simulink model "MCVT_Integration"  (R2020a)
settings: Solver=ode8  StartTime=0.0  StopTime=T_end  FixedStep=dt  RelTol=1e-3  EnableMultiTasking=on
values from neighbouring files (symbols this model uses):
  assumed_power_voltage = 1.25e2    (= 125)    [MCVT_Input_File.m]    (also in MCVT_Run_QuickCheck.m)
  battery_power_toggle = 1    [MCVT_Input_File.m]    (also in MCVT_Run_QuickCheck.m)
  battery_trickle_charge_param = 0.1    [MCVT_Input_File.m]    (also in MCVT_Run_QuickCheck.m)
  battery_trickle_leakage_param = 0.01    [MCVT_Input_File.m]    (also in MCVT_Run_QuickCheck.m)
  damage_case_id = 3    [MCVT_Input_File.m]    (also in MCVT_Run_QuickCheck.m = 1)
  dt = 1/fs    (= 0.001)    [MCVT_Run_QuickCheck.m]    (also in MCVT_Sim_Set.m)
  dt_2 = 1/fs_2    (= 0.001)    [MCVT_Run_QuickCheck.m]    (also in MCVT_Sim_Set.m)
  dt_3 = 1/fs_3    (= 0.001)    [MCVT_Run_QuickCheck.m]    (also in MCVT_Sim_Set.m)
  dt_5 = 1/fs_5    (= 0.005)    [MCVT_Run_QuickCheck.m]    (also in MCVT_Sim_Set.m)
  dt_8 = 1/fs_8    (= 0.05)    [MCVT_Run_QuickCheck.m]    (also in MCVT_Sim_Set.m)
  dt_9 = 1/fs_9    (= 0.001)    [MCVT_Run_QuickCheck.m]    (also in MCVT_Sim_Set.m)
  dust_met_duration = 3    [MCVT_Input_File.m]    (also in MCVT_Run_QuickCheck.m = 2)
  ECLSS_indoor_set_temperature = 298.15    [MCVT_Input_File.m]    (also in MCVT_Run_QuickCheck.m)
  energy_store_max_o = 150    [MCVT_Input_File.m]    (also in MCVT_Run_QuickCheck.m)
  energy_store_o = 120    [MCVT_Input_File.m]    (also in MCVT_Run_QuickCheck.m)
  fs = 1000    [MCVT_Run_QuickCheck.m]    (also in MCVT_Sim_Set.m)
  gain_charge_param = 1    [MCVT_Input_File.m]    (also in MCVT_Run_QuickCheck.m)
  ground_temperature = 373    [MCVT_Habitat_Design_Input_File.m]    (also in MCVT_Run_QuickCheck.m)
  I_max_charge = 800    [MCVT_Input_File.m]    (also in MCVT_Run_QuickCheck.m)
  I_max_discharge = 800    [MCVT_Input_File.m]    (also in MCVT_Run_QuickCheck.m)
  initial_pressure = 1e5    (= 100000)    [MCVT_Input_File.m]    (also in MCVT_Run_QuickCheck.m)
  initial_temperature = 295    [MCVT_Input_File.m]    (also in MCVT_Run_QuickCheck.m)
  interior_leak_rate = 0.00415    [MCVT_Input_File.m]    (also in MCVT_Run_QuickCheck.m)
  launch_dust_accum_start_time = 10    [MCVT_Input_File.m]    (also in MCVT_Run_QuickCheck.m = 2)
  launch_dust_accum_stop_time = 12    [MCVT_Input_File.m]    (also in MCVT_Run_QuickCheck.m = 3)
  meteorite_impact_time = 2    [MCVT_Input_File.m]    (also in MCVT_Run_QuickCheck.m = 1)
  nuclear_power_toggle = 1    [MCVT_Input_File.m]    (also in MCVT_Run_QuickCheck.m)
  print_interval = 30    [MCVT_Input_File.m]
  SOC_lower_thres = 0.5    [MCVT_Input_File.m]    (also in MCVT_Run_QuickCheck.m)
  SOC_upper_thres = 0.9    [MCVT_Input_File.m]    (also in MCVT_Run_QuickCheck.m)
  solar_power_toggle = 1    [MCVT_Input_File.m]    (also in MCVT_Run_QuickCheck.m)
  space_temperature = 4    [MCVT_Habitat_Design_Input_File.m]    (also in MCVT_Run_QuickCheck.m)
  Start_angle = 90    [MCVT_Input_File.m]    (also in MCVT_Run_QuickCheck.m)
undefined symbols (not defined in the file or in its folder): A_flow_restrictor, ACTIVITY_LOOKUP_TABLE, air, Aref, Bmap, Bmap2, Bmap3, comms, DiscIntNeverNeededParam, DmgSPL, dust_parameters, ECLSS_dust_damage_repair_time, ECLSS_heater_capacity, ECLSS_IPS_restrictor, ECLSS_IPS_setpointPressure, ECLSS_paint_damage_repair_time, ECLSS_radiatorLoop_discreteNumber, ECLSS_Vst, emptyActivity, expected_heat_add, FI, habitat, HIEM2SMM, HIEM2STM, HIEMDamageElements, impact_numbering, initial_SPLT_damage_index, input_air, input_Cond, input_CP, input_discretization, input_EV, input_Evap, input_radiatorLoop, K_ECLSS_dust_single, K_ECLSS_paint_single, K_Structure_damage_single, Kref, kW_to_W, meteoroidDustDep, mFRF, ndelays, nominalDustDep, noverlap, nuclear_toggle_gain, output_limits, panel_paint0, Ps0, reference_temperature, RepairTime_ECLSS_factor, Sensor_Location_SMM, SMM_T0, SMM_T1, SMM_T2, SMM_T3, SMM_T4, SMM_T4_0, SMM_T4_1, SMM_T4_2, solar_FDD_K ... (+73)
libraries: neural x350, simulink x39, xpcutilitieslib x7, xpcrtudplib x7, dspstat3 x1
(part 32 of 43)

== system MCVT_Integration/Sys2 - Structural System/Sys2_Structural_System/Fault Detection and Diagnosis (FDD) with Binary Classifer/Subsystem/Enabled Subsystem3/Pattern Recognition Neural Network1/Layer 2 ==
  "a{1} " : Inport  PortDimensions=30  SampleTime=0.001
      mask Display: e = 0.06;c1 = [120 230 180]/255;c2 = ([120 230 180]+0)/(256+128);c3 = ([120 230 180]+128)/(256+128);patch([0 1 1 0],[0 0 1 1],c1);patch([0 1 1 1-e 1-e 0+e],[0 0 1 1-e 0+e 0+e],c2);patch([0 0 1 1-e 0+e 0+e],[0 1 1 1-e 1-e 0+e],c3);plot([0 1 1 0 0],[0 0 1 1 0]);
  Delays 1 : Subsystem
      mask Display: e = 0.06;c1 = [120 150 230]/255;c2 = ([120 150 230]+0)/383;c3 = ([120 150 230]+128)/383;patch([0 1 1 0],[0 0 1 1],c1);patch([0 1 1 1-e 1-e 0+e],[0 0 1 1-e 0+e 0+e],c2);patch([0 0 1 1-e 0+e 0+e],[0 1 1 1-e 1-e 0+e],c3);plot([0 1 1 0 0],[0 0 1 1 0]);disp('0');
  "LW{2,1}" : Subsystem
      mask Display: e = 0.06;c1 = [120 150 230]/255;c2 = ([120 150 230]+0)/383;c3 = ([120 150 230]+128)/383;patch([0 1 1 0],[0 0 1 1],c1);patch([0 1 1 1-e 1-e 0+e],[0 0 1 1-e 0+e 0+e],c2);patch([0 0 1 1-e 0+e 0+e],[0 1 1 1-e 1-e 0+e],c3);plot([0 1 1 0 0],[0 0 1 1 0]);disp('W');
  "b{2}" : Constant  Value=[-1.5675181578718626607127362149185501039028167724609375;-1.3718445952097766404875756052206270396709442138671875;1.0992311692557044100482244175509549677371978759765625;1.1150228436739049175940863278810866177082061767578125;0.85025790232238362964523048503906466066837310791015625;-0.6689209990231959057638277954538352787494659423828125;-0.54887147293178861762186215855763293802738189697265625;-0.43766829067725743218630896080867387354373931884765625;-0.208936085733707643807832710081129334867000579833984375;0.057764402550326297836047473310827626846730709075927734375;-0.1666376935928840230527470112065202556550502777099609375;0.278159185776559070291114039719104766845703125;0.372658163201040559808774332850589416921138763427734375;0.5401695979973555328257361907162703573703765869140625;-0.714168735853778269273561818408779799938201904296875;0.8295230504828852158283325479715131223201751708984375;0.95406994019251578631468646563007496297359466552734375;-1.2131549221309152475356540890061296522617340087890625;1.267271803453536183070582410437054932117462158203125;-1.4500424122772075374854239271371625363826751708984375]
      mask Display: e = 0.06;c1 = [120 150 230]/255;c2 = ([120 150 230]+0)/383;c3 = ([120 150 230]+128)/383;patch([0 1 1 0],[0 0 1 1],c1);patch([0 1 1 1-e 1-e 0+e],[0 0 1 1-e 0+e 0+e],c2);patch([0 0 1 1-e 0+e 0+e],[0 1 1 1-e 1-e 0+e],c3);plot([0 1 1 0 0],[0 0 1 1 0]);disp('b');
  netsum : Sum  Inputs=2  InputSameDT=on  SaturateOnIntegerOverflow=on  OutDataTypeStr=Inherit: Same as first input
      mask Display: e = 0.06;c1 = [120 150 230]/255;c2 = ([120 150 230]+0)/383;c3 = ([120 150 230]+128)/383;patch([0 1 1 0],[0 0 1 1],c1);patch([0 1 1 1-e 1-e 0+e],[0 0 1 1-e 0+e 0+e],c2);patch([0 0 1 1-e 0+e 0+e],[0 1 1 1-e 1-e 0+e],c3);plot([0 1 1 0 0],[0 0 1 1 0]);disp('+');
  tansig : neural/Transfer Functions/tansig
  "a{2}" : Outport  InitialOutput=[0;0;0;0;0;0;0;0;0;0;0;0;0;0;0;0;0;0;0;0]
      mask Display: e = 0.06;c1 = [120 230 180]/255;c2 = ([120 230 180]+0)/(256+128);c3 = ([120 230 180]+128)/(256+128);patch([0 1 1 0],[0 0 1 1],c1);patch([0 1 1 1-e 1-e 0+e],[0 0 1 1-e 0+e 0+e],c2);patch([0 0 1 1-e 0+e 0+e],[0 1 1 1-e 1-e 0+e],c3);plot([0 1 1 0 0],[0 0 1 1 0]);
  wires:
  "a{1} "/1 -> Delays 1/1("p{2}")
  Delays 1/1("pd{2,1}") -> "LW{2,1}"/1("ad{2,1}")
  "LW{2,1}"/1("lz{2,1}") -> netsum/1
  "b{2}"/1 -> netsum/2
  netsum/1 -> tansig/1
  tansig/1 -> "a{2}"/1

== system MCVT_Integration/Sys2 - Structural System/Sys2_Structural_System/Fault Detection and Diagnosis (FDD) with Binary Classifer/Subsystem/Enabled Subsystem3/Pattern Recognition Neural Network1/Layer 3 ==
  "a{2} " : Inport  PortDimensions=20  SampleTime=0.001
      mask Display: e = 0.06;c1 = [120 230 180]/255;c2 = ([120 230 180]+0)/(256+128);c3 = ([120 230 180]+128)/(256+128);patch([0 1 1 0],[0 0 1 1],c1);patch([0 1 1 1-e 1-e 0+e],[0 0 1 1-e 0+e 0+e],c2);patch([0 0 1 1-e 0+e 0+e],[0 1 1 1-e 1-e 0+e],c3);plot([0 1 1 0 0],[0 0 1 1 0]);
  Delays 1 : Subsystem
      mask Display: e = 0.06;c1 = [120 150 230]/255;c2 = ([120 150 230]+0)/383;c3 = ([120 150 230]+128)/383;patch([0 1 1 0],[0 0 1 1],c1);patch([0 1 1 1-e 1-e 0+e],[0 0 1 1-e 0+e 0+e],c2);patch([0 0 1 1-e 0+e 0+e],[0 1 1 1-e 1-e 0+e],c3);plot([0 1 1 0 0],[0 0 1 1 0]);disp('0');
  "LW{3,2}" : Subsystem
      mask Display: e = 0.06;c1 = [120 150 230]/255;c2 = ([120 150 230]+0)/383;c3 = ([120 150 230]+128)/383;patch([0 1 1 0],[0 0 1 1],c1);patch([0 1 1 1-e 1-e 0+e],[0 0 1 1-e 0+e 0+e],c2);patch([0 0 1 1-e 0+e 0+e],[0 1 1 1-e 1-e 0+e],c3);plot([0 1 1 0 0],[0 0 1 1 0]);disp('W');
  "b{3}" : Constant  Value=[1.461288307463145752507216457161121070384979248046875;1.158168800422737287902918978943489491939544677734375;0.82605315684820423971501668347627855837345123291015625;-0.4114092128700901351834318120381794869899749755859375;-0.397272642706039402771267532443744130432605743408203125;-0.16670204583020098443313372627017088234424591064453125;0.52460758887335756650571738646249286830425262451171875;-0.83480056037617156317054423197987489402294158935546875;-1.258774443942892506953512565814889967441558837890625;1.7012363464620114950065499215270392596721649169921875]
      mask Display: e = 0.06;c1 = [120 150 230]/255;c2 = ([120 150 230]+0)/383;c3 = ([120 150 230]+128)/383;patch([0 1 1 0],[0 0 1 1],c1);patch([0 1 1 1-e 1-e 0+e],[0 0 1 1-e 0+e 0+e],c2);patch([0 0 1 1-e 0+e 0+e],[0 1 1 1-e 1-e 0+e],c3);plot([0 1 1 0 0],[0 0 1 1 0]);disp('b');
  netsum : Sum  Inputs=2  InputSameDT=on  SaturateOnIntegerOverflow=on  OutDataTypeStr=Inherit: Same as first input
      mask Display: e = 0.06;c1 = [120 150 230]/255;c2 = ([120 150 230]+0)/383;c3 = ([120 150 230]+128)/383;patch([0 1 1 0],[0 0 1 1],c1);patch([0 1 1 1-e 1-e 0+e],[0 0 1 1-e 0+e 0+e],c2);patch([0 0 1 1-e 0+e 0+e],[0 1 1 1-e 1-e 0+e],c3);plot([0 1 1 0 0],[0 0 1 1 0]);disp('+');
  tansig : neural/Transfer Functions/tansig
  "a{3}" : Outport  InitialOutput=[0;0;0;0;0;0;0;0;0;0]
      mask Display: e = 0.06;c1 = [120 230 180]/255;c2 = ([120 230 180]+0)/(256+128);c3 = ([120 230 180]+128)/(256+128);patch([0 1 1 0],[0 0 1 1],c1);patch([0 1 1 1-e 1-e 0+e],[0 0 1 1-e 0+e 0+e],c2);patch([0 0 1 1-e 0+e 0+e],[0 1 1 1-e 1-e 0+e],c3);plot([0 1 1 0 0],[0 0 1 1 0]);
  wires:
  "a{2} "/1 -> Delays 1/1("p{3}")
  Delays 1/1("pd{3,2}") -> "LW{3,2}"/1("ad{3,2}")
  "LW{3,2}"/1("lz{3,2}") -> netsum/1
  "b{3}"/1 -> netsum/2
  netsum/1 -> tansig/1
  tansig/1 -> "a{3}"/1

== system MCVT_Integration/Sys2 - Structural System/Sys2_Structural_System/Fault Detection and Diagnosis (FDD) with Binary Classifer/Subsystem/Enabled Subsystem3/Pattern Recognition Neural Network1/Layer 4 ==
  "a{3} " : Inport  PortDimensions=10  SampleTime=0.001
      mask Display: e = 0.06;c1 = [120 230 180]/255;c2 = ([120 230 180]+0)/(256+128);c3 = ([120 230 180]+128)/(256+128);patch([0 1 1 0],[0 0 1 1],c1);patch([0 1 1 1-e 1-e 0+e],[0 0 1 1-e 0+e 0+e],c2);patch([0 0 1 1-e 0+e 0+e],[0 1 1 1-e 1-e 0+e],c3);plot([0 1 1 0 0],[0 0 1 1 0]);
  Delays 1 : Subsystem
      mask Display: e = 0.06;c1 = [120 150 230]/255;c2 = ([120 150 230]+0)/383;c3 = ([120 150 230]+128)/383;patch([0 1 1 0],[0 0 1 1],c1);patch([0 1 1 1-e 1-e 0+e],[0 0 1 1-e 0+e 0+e],c2);patch([0 0 1 1-e 0+e 0+e],[0 1 1 1-e 1-e 0+e],c3);plot([0 1 1 0 0],[0 0 1 1 0]);disp('0');
  "LW{4,3}" : Subsystem
      mask Display: e = 0.06;c1 = [120 150 230]/255;c2 = ([120 150 230]+0)/383;c3 = ([120 150 230]+128)/383;patch([0 1 1 0],[0 0 1 1],c1);patch([0 1 1 1-e 1-e 0+e],[0 0 1 1-e 0+e 0+e],c2);patch([0 0 1 1-e 0+e 0+e],[0 1 1 1-e 1-e 0+e],c3);plot([0 1 1 0 0],[0 0 1 1 0]);disp('W');
  "b{4}" : Constant  Value=[-0.08562532905421325890582551210172823630273342132568359375;0.8902934228766004043365001052734442055225372314453125;-0.8595801172941406331773350757430307567119598388671875;-0.1235614075244175769086041327682323753833770751953125;1.164751385274076067588566729682497680187225341796875]
      mask Display: e = 0.06;c1 = [120 150 230]/255;c2 = ([120 150 230]+0)/383;c3 = ([120 150 230]+128)/383;patch([0 1 1 0],[0 0 1 1],c1);patch([0 1 1 1-e 1-e 0+e],[0 0 1 1-e 0+e 0+e],c2);patch([0 0 1 1-e 0+e 0+e],[0 1 1 1-e 1-e 0+e],c3);plot([0 1 1 0 0],[0 0 1 1 0]);disp('b');
  netsum : Sum  Inputs=2  InputSameDT=on  SaturateOnIntegerOverflow=on  OutDataTypeStr=Inherit: Same as first input
      mask Display: e = 0.06;c1 = [120 150 230]/255;c2 = ([120 150 230]+0)/383;c3 = ([120 150 230]+128)/383;patch([0 1 1 0],[0 0 1 1],c1);patch([0 1 1 1-e 1-e 0+e],[0 0 1 1-e 0+e 0+e],c2);patch([0 0 1 1-e 0+e 0+e],[0 1 1 1-e 1-e 0+e],c3);plot([0 1 1 0 0],[0 0 1 1 0]);disp('+');
  softmax : neural/Transfer Functions/softmax
      settings: SourceProductBaseCode=NN
  "a{4}" : Outport  InitialOutput=[0;0;0;0;0]
      mask Display: e = 0.06;c1 = [120 230 180]/255;c2 = ([120 230 180]+0)/(256+128);c3 = ([120 230 180]+128)/(256+128);patch([0 1 1 0],[0 0 1 1],c1);patch([0 1 1 1-e 1-e 0+e],[0 0 1 1-e 0+e 0+e],c2);patch([0 0 1 1-e 0+e 0+e],[0 1 1 1-e 1-e 0+e],c3);plot([0 1 1 0 0],[0 0 1 1 0]);
  wires:
  "a{3} "/1 -> Delays 1/1("p{4}")
  Delays 1/1("pd{4,3}") -> "LW{4,3}"/1("ad{4,3}")
  "LW{4,3}"/1("lz{4,3}") -> netsum/1
  "b{4}"/1 -> netsum/2
  netsum/1 -> softmax/1
  softmax/1 -> "a{4}"/1

== system MCVT_Integration/Sys2 - Structural System/Sys2_Structural_System/Fault Detection and Diagnosis (FDD) with Binary Classifer/Subsystem/Enabled Subsystem3/Pattern Recognition Neural Network1/Process Input 1 ==
  x : Inport  PortDimensions=45  SampleTime=0.001
      mask Display: e = 0.06;c1 = [120 230 180]/255;c2 = ([120 230 180]+0)/(256+128);c3 = ([120 230 180]+128)/(256+128);patch([0 1 1 0],[0 0 1 1],c1);patch([0 1 1 1-e 1-e 0+e],[0 0 1 1-e 0+e 0+e],c2);patch([0 0 1 1-e 0+e 0+e],[0 1 1 1-e 1-e 0+e],c3);plot([0 1 1 0 0],[0 0 1 1 0]);
  mapminmax : neural/Processing Functions/mapminmax  xmin=[0;0;0;0;0;0;0;0;0;0.169206936522193485128440215703;0.217935425059106208101056267878;0.0691683929316798951081324275947;0.0272070524150397827223279989539;0.0520052755437293359741524056972;0.0202909509001970855823770989446;0.0391341679420866839222981070634;0.025851121817247000450601390753;0.00978196766557235496131195162661;1;1;1;1;1;1;1;1;1;0.169585455186831379759127003126;0.271662275988948098692787880282;0.067846889674121724222999318954;0.0403562897511558077434656865989;0.044720296174577733872013141081;0.0210397701282347276974249439263;0.0481979692634679421359145123915;0.0263534724010484021350375627435;0.0146758692068002585817687588587;1;1;1;1;1;1;1;1;1]  xmax=[10;10;10;10;10;10;10;10;10;1;1;1;1;0.612543655466822656308067962527;0.567638403365003041400882466405;1;1;1;96;274;98;95;96;327;20;95;86;1;1;1;1;0.605289397240143633993625371659;0.582993139346640432130186582071;1;0.911474519440508168521830612008;1;96;274;98;95;96;327;20;95;86]  ymin=-1  ymax=1
  p : Outport  PortDimensions=45  SampleTime=0.001
      mask Display: e = 0.06;c1 = [120 230 180]/255;c2 = ([120 230 180]+0)/(256+128);c3 = ([120 230 180]+128)/(256+128);patch([0 1 1 0],[0 0 1 1],c1);patch([0 1 1 1-e 1-e 0+e],[0 0 1 1-e 0+e 0+e],c2);patch([0 0 1 1-e 0+e 0+e],[0 1 1 1-e 1-e 0+e],c3);plot([0 1 1 0 0],[0 0 1 1 0]);
  wires:
  x/1 -> mapminmax/1
  mapminmax/1 -> p/1

== system MCVT_Integration/Sys2 - Structural System/Sys2_Structural_System/Fault Detection and Diagnosis (FDD) with Binary Classifer/Subsystem/Enabled Subsystem3/Pattern Recognition Neural Network1/Process Output 1 ==
  a : Inport  PortDimensions=5  SampleTime=0.001
      mask Display: e = 0.06;c1 = [120 230 180]/255;c2 = ([120 230 180]+0)/(256+128);c3 = ([120 230 180]+128)/(256+128);patch([0 1 1 0],[0 0 1 1],c1);patch([0 1 1 1-e 1-e 0+e],[0 0 1 1-e 0+e 0+e],c2);patch([0 0 1 1-e 0+e 0+e],[0 1 1 1-e 1-e 0+e],c3);plot([0 1 1 0 0],[0 0 1 1 0]);
  y : Outport  PortDimensions=5  SampleTime=0.001
      mask Display: e = 0.06;c1 = [120 230 180]/255;c2 = ([120 230 180]+0)/(256+128);c3 = ([120 230 180]+128)/(256+128);patch([0 1 1 0],[0 0 1 1],c1);patch([0 1 1 1-e 1-e 0+e],[0 0 1 1-e 0+e 0+e],c2);patch([0 0 1 1-e 0+e 0+e],[0 1 1 1-e 1-e 0+e],c3);plot([0 1 1 0 0],[0 0 1 1 0]);
  wires:
  a/1 -> y/1

== system MCVT_Integration/Sys2 - Structural System/Sys2_Structural_System/Structural Thermal Model 2.2/STM Temperature Sensors/ Resistance Temperature Detector/Switch ON//OFF Control Block/Switch OFF ==
  In1 : Inport
  Clock1 : Clock
  MATLAB Function1 : MATLAB Function  TreatAsAtomicUnit=on
  No Signal : Scope
  OFF : Outport  VectorParamsAs1DForOutWhenUnconnected=off
  wires:
  In1/1 -> MATLAB Function1/1(u)
  Clock1/1 -> MATLAB Function1/2(Time)
  MATLAB Function1/1(y) -> OFF/1, No Signal/1

== system MCVT_Integration/Sys2 - Structural System/Sys2_Structural_System/Structural Thermal Model 2.2/STM Temperature Sensors/ Resistance Temperature Detector/Switch ON//OFF Control Block/Switch ON ==
  In1 : Inport
  Gain2 : Gain
  Signal Received : Scope
  ON : Outport  VectorParamsAs1DForOutWhenUnconnected=off
  wires:
  Gain2/1 -> ON/1, Signal Received/1
  In1/1 -> Gain2/1

== system MCVT_Integration/Sys2 - Structural System/Sys2_Structural_System/Structural Thermal Model 2.2/STM Temperature Sensors/ Resistance Temperature Detector1/Switch ON//OFF Control Block/Switch OFF ==
  In1 : Inport
  Clock1 : Clock
  MATLAB Function1 : MATLAB Function  TreatAsAtomicUnit=on
  No Signal : Scope
  OFF : Outport  VectorParamsAs1DForOutWhenUnconnected=off
  wires:
  MATLAB Function1/1(y) -> No Signal/1, OFF/1
  Clock1/1 -> MATLAB Function1/2(Time)
  In1/1 -> MATLAB Function1/1(u)

== system MCVT_Integration/Sys2 - Structural System/Sys2_Structural_System/Structural Thermal Model 2.2/STM Temperature Sensors/ Resistance Temperature Detector1/Switch ON//OFF Control Block/Switch ON ==
  In1 : Inport
  Gain2 : Gain
  Signal Received : Scope
  ON : Outport  VectorParamsAs1DForOutWhenUnconnected=off
  wires:
  In1/1 -> Gain2/1
  Gain2/1 -> Signal Received/1, ON/1

== system MCVT_Integration/Sys2 - Structural System/Sys2_Structural_System/Structural Thermal Model 2.2/STM Temperature Sensors/ Resistance Temperature Detector2/Switch ON//OFF Control Block/Switch OFF ==
  In1 : Inport
  Clock1 : Clock
  MATLAB Function1 : MATLAB Function  TreatAsAtomicUnit=on
  No Signal : Scope
  OFF : Outport  VectorParamsAs1DForOutWhenUnconnected=off
  wires:
  In1/1 -> MATLAB Function1/1(u)
  Clock1/1 -> MATLAB Function1/2(Time)
  MATLAB Function1/1(y) -> OFF/1, No Signal/1

== system MCVT_Integration/Sys2 - Structural System/Sys2_Structural_System/Structural Thermal Model 2.2/STM Temperature Sensors/ Resistance Temperature Detector2/Switch ON//OFF Control Block/Switch ON ==
  In1 : Inport
  Gain2 : Gain
  Signal Received : Scope
  ON : Outport  VectorParamsAs1DForOutWhenUnconnected=off
  wires:
  Gain2/1 -> ON/1, Signal Received/1
  In1/1 -> Gain2/1

== system MCVT_Integration/Sys3 - Power System/Power Generation Subsystem1/Nuclear Power Generation/SP-100 Power Generation/	Power Generation/Interpolation/Four Converters Dust Disturbance interp ==
  dust : Inport
  flow : Inport  Port=2
  temp flux : Inport  Port=3
  Bias : Bias  Bias=1
  Divide : Product  Inputs=*/
  Nominal Heat Transfer Rate Passed to 1 Stirling Converter1 : Constant  Value=expected_heat_add
  Product1 : Product
  Product2 : Product
  sec coolant flowrate*specific heat of coolant1 : Gain  Gain=sub3_flowrate_2nd_coolant*sub3_spec_heat_2nd_loop
  sub3_numb_converters : Gain  Gain=sub3_num_converters
  sec_loop_drop : Outport
  wires:
  sec coolant flowrate*specific heat of coolant1/1 -> Divide/2
  sub3_numb_converters/1 -> sec_loop_drop/1
  Product1/1 -> Divide/1
  Nominal Heat Transfer Rate Passed to 1 Stirling Converter1/1 -> Product1/1
  dust/1 -> Product1/2
  Divide/1 -> Product2/1
  Product2/1 -> sub3_numb_converters/1
  temp flux/1 -> Bias/1
  Bias/1 -> Product2/2
  flow/1 -> sec coolant flowrate*specific heat of coolant1/1

== system MCVT_Integration/Sys3 - Power System/Power Generation Subsystem1/Nuclear Power Generation/SP-100 Power Generation/	Power Generation/Interpolation/Four Converters Dust Disturbance low ==
  dust : Inport
  flow : Inport  Port=2
  temp flux : Inport  Port=3
  Bias : Bias  Bias=1
  Divide : Product  Inputs=*/
  Nominal Heat Transfer Rate Passed to 1 Stirling Converter1 : Constant  Value=expected_heat_add
  Product1 : Product
  Product2 : Product
  sec coolant flowrate*specific heat of coolant1 : Gain  Gain=sub3_flowrate_2nd_coolant*sub3_spec_heat_2nd_loop
  sub3_numb_converters : Gain  Gain=sub3_num_converters
  sec_loop_drop : Outport
  wires:
  sec coolant flowrate*specific heat of coolant1/1 -> Divide/2
  sub3_numb_converters/1 -> sec_loop_drop/1
  Product1/1 -> Divide/1
  Nominal Heat Transfer Rate Passed to 1 Stirling Converter1/1 -> Product1/1
  dust/1 -> Product1/2
  Divide/1 -> Product2/1
  Product2/1 -> sub3_numb_converters/1
  temp flux/1 -> Bias/1
  Bias/1 -> Product2/2
  flow/1 -> sec coolant flowrate*specific heat of coolant1/1

== system MCVT_Integration/Sys3 - Power System/Power Generation Subsystem1/Nuclear Power Generation/SP-100 Power Generation/	Power Generation/Interpolation/Four Converters Dust Disturbance up ==
  dust : Inport
  flow : Inport  Port=2
  temp flux : Inport  Port=3
  Bias : Bias  Bias=1
  Divide : Product  Inputs=*/
  Nominal Heat Transfer Rate Passed to 1 Stirling Converter1 : Constant  Value=expected_heat_add
  Product1 : Product
  Product2 : Product
  sec coolant flowrate*specific heat of coolant1 : Gain  Gain=sub3_flowrate_2nd_coolant*sub3_spec_heat_2nd_loop
  sub3_numb_converters : Gain  Gain=sub3_num_converters
  sec_loop_drop : Outport
  wires:
  sec coolant flowrate*specific heat of coolant1/1 -> Divide/2
  sub3_numb_converters/1 -> sec_loop_drop/1
  Product1/1 -> Divide/1
  Nominal Heat Transfer Rate Passed to 1 Stirling Converter1/1 -> Product1/1
  dust/1 -> Product1/2
  Divide/1 -> Product2/1
  Product2/1 -> sub3_numb_converters/1
  temp flux/1 -> Bias/1
  Bias/1 -> Product2/2
  flow/1 -> sec coolant flowrate*specific heat of coolant1/1

== system MCVT_Integration/Sys3 - Power System/Power Generation Subsystem1/Nuclear Power Generation/SP-100 Power Generation/	Power Generation/Interpolation/Secondary Loop + Stirling Loop interp ==
  Tc2 (K) : Inport
  Flow : Inport  Port=2
  sec_loop_drop : Inport  Port=3
  Constant : Constant  Value=sub3_cyceff
  IC1 : InitialCondition  Value=sub3_init_tc2
  MATLAB Function1 : MATLAB Function  TreatAsAtomicUnit=on
  Product : Product  Inputs=3
  Subtract : Sum  Inputs=+-
  sec coolant flowrate*specific heat of coolant1 : Gain  Gain=sub3_flowrate_2nd_coolant*sub3_spec_heat_2nd_loop
  Tc1 (K) : Outport
  Power Out (kW) : Outport  Port=2
  wires:
  Subtract/1 -> Tc1 (K)/1
  sec_loop_drop/1 -> Subtract/2, Product/1  "sec_loop_drop"
  Flow/1 -> sec coolant flowrate*specific heat of coolant1/1
  sec coolant flowrate*specific heat of coolant1/1 -> Product/2
  Constant/1 -> Product/3
  Product/1 -> Power Out (kW)/1
  Tc2 (K)/1 -> MATLAB Function1/1(u)
  MATLAB Function1/1(Tc2_lower) -> IC1/1
  IC1/1 -> Subtract/1

== system MCVT_Integration/Sys3 - Power System/Power Generation Subsystem1/Nuclear Power Generation/SP-100 Power Generation/	Power Generation/Interpolation/Secondary Loop + Stirling Loop lower ==
  Tc2 (K) : Inport
  Flow : Inport  Port=2
  sec_loop_drop : Inport  Port=3
  Constant : Constant  Value=sub3_cyceff
  IC1 : InitialCondition  Value=sub3_init_tc2
  MATLAB Function1 : MATLAB Function  TreatAsAtomicUnit=on
  Product : Product  Inputs=3
  Subtract : Sum  Inputs=+-
  sec coolant flowrate*specific heat of coolant1 : Gain  Gain=sub3_flowrate_2nd_coolant*sub3_spec_heat_2nd_loop
  Tc1 (K) : Outport
  Power Out (kW) : Outport  Port=2
  wires:
  Subtract/1 -> Tc1 (K)/1
  sec_loop_drop/1 -> Product/1, Subtract/2  "sec_loop_drop"
  Flow/1 -> sec coolant flowrate*specific heat of coolant1/1
  sec coolant flowrate*specific heat of coolant1/1 -> Product/2
  Constant/1 -> Product/3
  Product/1 -> Power Out (kW)/1
  Tc2 (K)/1 -> MATLAB Function1/1(u)
  MATLAB Function1/1(Tc2_lower) -> IC1/1
  IC1/1 -> Subtract/1

== system MCVT_Integration/Sys3 - Power System/Power Generation Subsystem1/Nuclear Power Generation/SP-100 Power Generation/	Power Generation/Interpolation/Secondary Loop + Stirling Loop upper ==
  Tc2 (K) : Inport
  Flow : Inport  Port=2
  sec_loop_drop : Inport  Port=3
  Constant : Constant  Value=sub3_cyceff
  IC1 : InitialCondition  Value=sub3_init_tc2
  MATLAB Function1 : MATLAB Function  TreatAsAtomicUnit=on
  Product : Product  Inputs=3
  Subtract : Sum  Inputs=+-
  sec coolant flowrate*specific heat of coolant1 : Gain  Gain=sub3_flowrate_2nd_coolant*sub3_spec_heat_2nd_loop
  Tc1 (K) : Outport
  Power Out (kW) : Outport  Port=2
  wires:
  Subtract/1 -> Tc1 (K)/1
  sec_loop_drop/1 -> Subtract/2, Product/1  "sec_loop_drop"
  Flow/1 -> sec coolant flowrate*specific heat of coolant1/1
  sec coolant flowrate*specific heat of coolant1/1 -> Product/2
  Constant/1 -> Product/3
  Product/1 -> Power Out (kW)/1
  Tc2 (K)/1 -> MATLAB Function1/1(u)
  MATLAB Function1/1(Tc2_lower) -> IC1/1
  IC1/1 -> Subtract/1

== system MCVT_Integration/Sys3 - Power System/Power Generation Subsystem1/Nuclear Power Generation/SP-100 Power Generation/	Power Generation/Nominal Power/Four Converters Dust Disturbance ==
  dust : Inport
  flow : Inport  Port=2
  temp flux : Inport  Port=3
  Bias : Bias  Bias=1
  Divide : Product  Inputs=*/
  Nominal Heat Transfer Rate Passed to 1 Stirling Converter1 : Constant  Value=expected_heat_add
  Product1 : Product
  Product2 : Product
  sec coolant flowrate*specific heat of coolant1 : Gain  Gain=sub3_flowrate_2nd_coolant*sub3_spec_heat_2nd_loop
  sub3_numb_converters : Gain  Gain=sub3_num_converters
  sec_loop_drop : Outport
  wires:
  sec coolant flowrate*specific heat of coolant1/1 -> Divide/2
  sub3_numb_converters/1 -> sec_loop_drop/1
  Product1/1 -> Divide/1
  Nominal Heat Transfer Rate Passed to 1 Stirling Converter1/1 -> Product1/1
  dust/1 -> Product1/2
  Divide/1 -> Product2/1
  Product2/1 -> sub3_numb_converters/1
  temp flux/1 -> Bias/1
  Bias/1 -> Product2/2
  flow/1 -> sec coolant flowrate*specific heat of coolant1/1

== system MCVT_Integration/Sys3 - Power System/Power Generation Subsystem1/Nuclear Power Generation/SP-100 Power Generation/	Power Generation/Nominal Power/Secondary Loop + Stirling Loop ==
  y_process_scale_lower : Inport
  Flow : Inport  Port=2
  sec_loop_drop : Inport  Port=3
  Constant : Constant  Value=sub3_cyceff
  IC1 : InitialCondition  Value=sub3_init_tc2
  MATLAB Function1 : MATLAB Function  TreatAsAtomicUnit=on
  Product : Product  Inputs=3
  Subtract : Sum  Inputs=+-
  sec coolant flowrate*specific heat of coolant1 : Gain  Gain=sub3_flowrate_2nd_coolant*sub3_spec_heat_2nd_loop
  Tc1_lower : Outport
  Power Out : Outport  Port=2
  wires:
  Subtract/1 -> Tc1_lower/1
  sec_loop_drop/1 -> Subtract/2, Product/1  "sec_loop_drop_nom"
  Flow/1 -> sec coolant flowrate*specific heat of coolant1/1
  sec coolant flowrate*specific heat of coolant1/1 -> Product/2
  Constant/1 -> Product/3
  Product/1 -> Power Out/1
  y_process_scale_lower/1 -> MATLAB Function1/1(u)
  MATLAB Function1/1(Tc2_lower) -> IC1/1
  IC1/1 -> Subtract/1

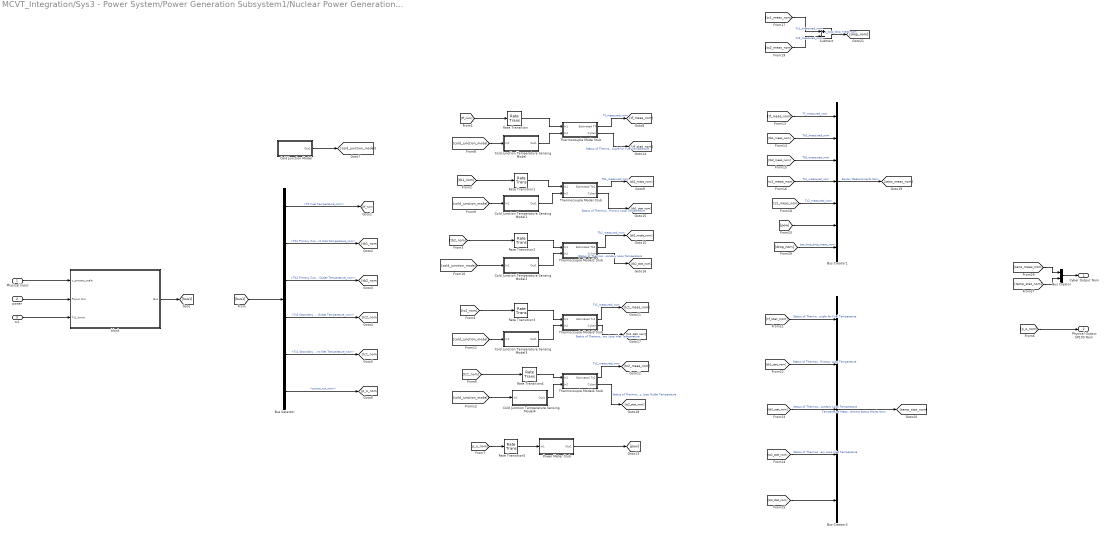
== system MCVT_Integration/Sys3 - Power System/Power Generation Subsystem1/Nuclear Power Generation/SP-100 Power Generation/	Power Generation/Nominal Power/Sensor Models ==
  Physical Input : Inport
  power : Inport  Port=2
  tc1 : Inport  Port=3
  Bus Creator : BusCreator  Inputs=2
  Bus Creator1 : BusCreator  Inputs=7
  Bus Creator3 : BusCreator  Inputs=5
  Bus Selector : BusSelector  OutputSignals=TF Fuel Temperature_nom,Tb1 Primary Coolant Inlet Temperature_nom,Tb2 Primary Coolant Outlet Temperature_nom,Tc2 Secondary Coolant Outlet Temperature_nom,Tc1 Secondary Coolant Inlet Temperature_nom,power_out_nom
  Cold Junction Model : Subsystem
  Cold Junction Temperature Sensing Model : Subsystem
  Cold Junction Temperature Sensing Model1 : Subsystem
  Cold Junction Temperature Sensing Model2 : Subsystem
  Cold Junction Temperature Sensing Model3 : Subsystem
  Cold Junction Temperature Sensing Model4 : Subsystem
  Power Meter Stub : Subsystem
  Rate Transition{,1..5} (6 alike) : RateTransition  OutPortSampleTime=dt_3
  Subtract : Sum  Inputs=+-
  Thermocouple Mode Stub : Subsystem
  Thermocouple Model Stub : Subsystem
  Thermocouple Model2 Stub : Subsystem
  Thermocouple Model3 Stub : Subsystem
  Thermocouple Model4 Stub : Subsystem
  block : Subsystem
  Cyber Output Nom : Outport
  Physical Output SP100 Nom : Outport  Port=2
  wires:
  [tf_nom] -> Rate Transition/1
  [tf_meas_nom] -> Bus Creator1/1  "Tf_measured_nom"
  Cold Junction Temperature Sensing Model/1(Out1) -> Thermocouple Mode Stub/2(In2)
  [tb1_meas_nom] -> Bus Creator1/2  "Tb1_measured_nom"
  [tb1_nom] -> Rate Transition1/1
  [tb2_meas_nom] -> Bus Creator1/3  "Tb2_measured_nom"
  [tb2_nom] -> Rate Transition2/1
  [cold_junction_model] -> Cold Junction Temperature Sensing Model/1(In1)
  Cold Junction Temperature Sensing Model2/1(Out1) -> Thermocouple Model2 Stub/2(In2)
  Cold Junction Temperature Sensing Model1/1(Out1) -> Thermocouple Model Stub/2(In2)
  Cold Junction Temperature Sensing Model4/1(Out1) -> Thermocouple Model4 Stub/2(In2)
  Cold Junction Temperature Sensing Model3/1(Out1) -> Thermocouple Model3 Stub/2(In2)
  Bus Creator1/1 -> [sens_meas_nom]  "Sensor Measurements Nom"
  [tc1_meas_nom] -> Bus Creator1/4  "Tc1_measured_nom"
  [tc2_meas_nom] -> Bus Creator1/5  "Tc2_measured_nom"
  Bus Creator/1 -> Cyber Output Nom/1
  block/1(Bus) -> [bus1]
  [tc1_nom] -> Rate Transition4/1
  [tf_stat_nom] -> Bus Creator3/1  "Status of Thermocouple for Fuel Temperature"
  [tb1_stat_nom] -> Bus Creator3/2  "Status of Thermocouple for Primary Loop Temperature"
  [tb2_stat_nom] -> Bus Creator3/3  "Status of Thermocouple for Avg. Secondary Loop Temperature"
  [tc2_stat_nom] -> Bus Creator3/4  "Status of Thermocouple for Secondary Loop Inlet Temperature"
  Thermocouple Model4 Stub/2(Cyber) -> [tc2_stat_nom]  "Status of Thermocouple for Secondary Loop Outlet Temperature"
  Bus Creator3/1 -> [temp_stat_nom]  "Temperature Measurement Status Words Nom"
  [drop_nom] -> Bus Creator1/7  "sec_loop_drop_meas_nom"
  [pow] -> Bus Creator1/6
  Physical Input/1 -> block/1(y_process_scale)
  [tc2_nom] -> Rate Transition3/1
  Rate Transition/1 -> Thermocouple Mode Stub/1(In1)
  Rate Transition1/1 -> Thermocouple Model Stub/1(In1)
  Rate Transition2/1 -> Thermocouple Model2 Stub/1(In1)
  Rate Transition3/1 -> Thermocouple Model3 Stub/1(In1)
  Rate Transition4/1 -> Thermocouple Model4 Stub/1(In1)
  Rate Transition5/1 -> Power Meter Stub/1(In1)
  [p_o_nom] -> Physical Output SP100 Nom/1
  power/1 -> block/2(Power Out)
  tc1/1 -> block/3(Tc1_lower)
  [bus1] -> Bus Selector/1
  Bus Selector/1 -> [tf_nom]  "<TF Fuel Temperature_nom>"
  Bus Selector/2 -> [tb1_nom]  "<Tb1 Primary Coolant Inlet Temperature_nom>"
  Bus Selector/3 -> [tb2_nom]  "<Tb2 Primary Coolant Outlet Temperature_nom>"
  Bus Selector/4 -> [tc2_nom]  "<Tc2 Secondary Coolant Outlet Temperature_nom>"
  Bus Selector/5 -> [tc1_nom]  "<Tc1 Secondary Coolant Inlet Temperature_nom>"
  Bus Selector/6 -> [p_o_nom]  "<power_out_nom>"
  [p_o_nom] -> Rate Transition5/1
  Cold Junction Model/1(Out1) -> [cold_junction_model]
  [cold_junction_model] -> Cold Junction Temperature Sensing Model1/1(In1)
  [cold_junction_model] -> Cold Junction Temperature Sensing Model2/1(In1)
  [cold_junction_model] -> Cold Junction Temperature Sensing Model3/1(In1)
  [cold_junction_model] -> Cold Junction Temperature Sensing Model4/1(In1)
  Thermocouple Mode Stub/1(Estimated Tf) -> [tf_meas_nom]  "Tf_measured_nom"
  Thermocouple Model Stub/1(Estimated Tb1) -> [tb1_meas_nom]  "Tb1_measured_nom"
  Thermocouple Model2 Stub/1(Estimated Tb2) -> [tb2_meas_nom]  "Tb2_measured_nom"
  Thermocouple Model3 Stub/1(Estimated Tc1) -> [tc1_meas_nom]  "Tc1_measured_nom"
  [tc1_meas_nom] -> Subtract/1  "Tc1_measured_nom"
  Thermocouple Model4 Stub/1(Estimated Tc2) -> [tc2_meas_nom]  "Tc2_measured_nom"
  [tc2_meas_nom] -> Subtract/2  "Tc2_measured_nom"
  Power Meter Stub/1(Out1) -> [pow]
  Thermocouple Mode Stub/2(Cyber) -> [tf_stat_nom]  "Status of Thermocouple for Fuel Temperature"
  Thermocouple Model Stub/2(Cyber) -> [tb1_stat_nom]  "Status of Thermocouple for Primary Loop Temperature"
  Thermocouple Model2 Stub/2(Cyber) -> [tb2_stat_nom]  "Status of Thermocouple for Avg. Secondary Loop Temperature"
  Thermocouple Model3 Stub/2(Cyber) -> [tc1_stat_nom]  "Status of Thermocouple for Secondary Loop Inlet Temperature"
  [tc2_stat_nom] -> Bus Creator3/5
  [sens_meas_nom] -> Bus Creator/1
  [temp_stat_nom] -> Bus Creator/2
  Subtract/1 -> [drop_nom]  "sec_loop_drop_meas_nom"

== system MCVT_Integration/Sys3 - Power System/Power Generation Subsystem1/Nuclear Power Generation/SP-100 Power Generation/	Power Generation/Power Generation/Four Converters Dust Disturbance ==
  dust : Inport
  flow : Inport  Port=2
  temp flux : Inport  Port=3
  Bias : Bias  Bias=1
  Divide : Product  Inputs=*/
  Nominal Heat Transfer Rate Passed to 1 Stirling Converter : Constant  Value=expected_heat_add
  Product1 : Product
  Product2 : Product
  sec coolant flowrate*specific heat of coolant : Gain  Gain=sub3_flowrate_2nd_coolant*sub3_spec_heat_2nd_loop
  sub3_num_converters : Gain  Gain=sub3_num_converters
  sec_loop_drop : Outport
  wires:
  sec coolant flowrate*specific heat of coolant/1 -> Divide/2
  sub3_num_converters/1 -> sec_loop_drop/1
  Product1/1 -> Divide/1
  Nominal Heat Transfer Rate Passed to 1 Stirling Converter/1 -> Product1/1
  dust/1 -> Product1/2
  Divide/1 -> Product2/1
  Product2/1 -> sub3_num_converters/1
  temp flux/1 -> Bias/1
  Bias/1 -> Product2/2
  flow/1 -> sec coolant flowrate*specific heat of coolant/1

== system MCVT_Integration/Sys3 - Power System/Power Generation Subsystem1/Nuclear Power Generation/SP-100 Power Generation/	Power Generation/Power Generation/Heat Rejection1 ==
  dust_accumulation_in mg/cm^2 : Inport
  MATLAB Function : MATLAB Function  TreatAsAtomicUnit=on
  sub3_nuc_dust_convert : Gain  Gain=sub3_nuc_dust_convert
  Heat Rejection Fraction : Outport
  wires:
  dust_accumulation_in mg/cm^2/1 -> sub3_nuc_dust_convert/1  "dust_accum1"
  MATLAB Function/1(HRF) -> Heat Rejection Fraction/1
  sub3_nuc_dust_convert/1 -> MATLAB Function/1(dust_ratio)  "dust_accum2"

== system MCVT_Integration/Sys3 - Power System/Power Generation Subsystem1/Nuclear Power Generation/SP-100 Power Generation/	Power Generation/Power Generation/Secondary Loop + Stirling Loop ==
  y_process_scale_lower : Inport
  Flow : Inport  Port=2
  sec_loop_drop : Inport  Port=3
  Constant : Constant  Value=sub3_cyceff
  IC1 : InitialCondition  Value=sub3_init_tc2
  MATLAB Function1 : MATLAB Function  TreatAsAtomicUnit=on
  Product : Product  Inputs=3
  Subtract : Sum  Inputs=+-
  sec coolant flowrate*specific heat of coolant : Gain  Gain=sub3_flowrate_2nd_coolant*sub3_spec_heat_2nd_loop
  Tc1_lower : Outport
  Power Out : Outport  Port=2
  wires:
  Subtract/1 -> Tc1_lower/1
  sec_loop_drop/1 -> Subtract/2, Product/1  "sec_loop_drop"
  Flow/1 -> sec coolant flowrate*specific heat of coolant/1
  sec coolant flowrate*specific heat of coolant/1 -> Product/2
  Constant/1 -> Product/3
  Product/1 -> Power Out/1
  y_process_scale_lower/1 -> MATLAB Function1/1(u)
  MATLAB Function1/1(Tc2_lower) -> IC1/1
  IC1/1 -> Subtract/1

== system MCVT_Integration/Sys3 - Power System/Power Generation Subsystem1/Nuclear Power Generation/SP-100 Power Generation/	Power Generation/Sensor Models/Cold Junction Model ==
  Cold Junction Temperature : Constant
  Kelvin to Celcuis : Bias  Bias=-273.15
  Out1 : Outport
  wires:
  Cold Junction Temperature/1 -> Kelvin to Celcuis/1
  Kelvin to Celcuis/1 -> Out1/1

== system MCVT_Integration/Sys3 - Power System/Power Generation Subsystem1/Nuclear Power Generation/SP-100 Power Generation/	Power Generation/Sensor Models/Cold Junction Temperature Sensing Model ==
  note: Switch Control
      | 1 - Switch ON
      | 2 - Switch OFF
  In1 : Inport
  " Resistance Temperature Detector" : Subsystem
  " Switch ON/OFF Control Number" : Constant
  Celcuis to Kelvin : Bias  Bias=273.15
  Out1 : Outport
  wires:
  In1/1 -> " Resistance Temperature Detector"/1(Physical Interdependency - IN)
  " Switch ON/OFF Control Number"/1 -> " Resistance Temperature Detector"/2(Switch Control)
  Celcuis to Kelvin/1 -> Out1/1
  " Resistance Temperature Detector"/2(Measured Temperature) -> Celcuis to Kelvin/1  "Measured Temperature"

== system MCVT_Integration/Sys3 - Power System/Power Generation Subsystem1/Nuclear Power Generation/SP-100 Power Generation/	Power Generation/Sensor Models/Cold Junction Temperature Sensing Model1 ==
  note: Switch Control
      | 1 - Switch ON
      | 2 - Switch OFF
  In1 : Inport
  " Resistance Temperature Detector" : Subsystem
  " Switch ON/OFF Control Number" : Constant
  Celcuis to Kelvin : Bias  Bias=273.15
  Out1 : Outport
  wires:
  In1/1 -> " Resistance Temperature Detector"/1(Physical Interdependency - IN)
  " Switch ON/OFF Control Number"/1 -> " Resistance Temperature Detector"/2(Switch Control)
  Celcuis to Kelvin/1 -> Out1/1
  " Resistance Temperature Detector"/2(Measured Temperature) -> Celcuis to Kelvin/1  "Measured Temperature"

== system MCVT_Integration/Sys3 - Power System/Power Generation Subsystem1/Nuclear Power Generation/SP-100 Power Generation/	Power Generation/Sensor Models/Cold Junction Temperature Sensing Model2 ==
  note: Switch Control
      | 1 - Switch ON
      | 2 - Switch OFF
  In1 : Inport
  " Resistance Temperature Detector" : Subsystem
  " Switch ON/OFF Control Number" : Constant
  Celcuis to Kelvin : Bias  Bias=273.15
  Out1 : Outport
  wires:
  " Resistance Temperature Detector"/2(Measured Temperature) -> Celcuis to Kelvin/1  "Measured Temperature"
  Celcuis to Kelvin/1 -> Out1/1
  " Switch ON/OFF Control Number"/1 -> " Resistance Temperature Detector"/2(Switch Control)
  In1/1 -> " Resistance Temperature Detector"/1(Physical Interdependency - IN)

== system MCVT_Integration/Sys3 - Power System/Power Generation Subsystem1/Nuclear Power Generation/SP-100 Power Generation/	Power Generation/Sensor Models/Cold Junction Temperature Sensing Model3 ==
  note: Switch Control
      | 1 - Switch ON
      | 2 - Switch OFF
  In1 : Inport
  " Resistance Temperature Detector" : Subsystem
  " Switch ON/OFF Control Number" : Constant
  Celcuis to Kelvin : Bias  Bias=273.15
  Out1 : Outport
  wires:
  In1/1 -> " Resistance Temperature Detector"/1(Physical Interdependency - IN)
  " Switch ON/OFF Control Number"/1 -> " Resistance Temperature Detector"/2(Switch Control)
  Celcuis to Kelvin/1 -> Out1/1
  " Resistance Temperature Detector"/2(Measured Temperature) -> Celcuis to Kelvin/1  "Measured Temperature"

== system MCVT_Integration/Sys3 - Power System/Power Generation Subsystem1/Nuclear Power Generation/SP-100 Power Generation/	Power Generation/Sensor Models/Cold Junction Temperature Sensing Model4 ==
  note: Switch Control
      | 1 - Switch ON
      | 2 - Switch OFF
  In1 : Inport
  " Resistance Temperature Detector" : Subsystem
  " Switch ON/OFF Control Number" : Constant
  Celcuis to Kelvin : Bias  Bias=273.15
  Out1 : Outport
  wires:
  " Resistance Temperature Detector"/2(Measured Temperature) -> Celcuis to Kelvin/1  "Measured Temperature"
  Celcuis to Kelvin/1 -> Out1/1
  " Switch ON/OFF Control Number"/1 -> " Resistance Temperature Detector"/2(Switch Control)
  In1/1 -> " Resistance Temperature Detector"/1(Physical Interdependency - IN)

== system MCVT_Integration/Sys3 - Power System/Power Generation Subsystem1/Nuclear Power Generation/SP-100 Power Generation/	Power Generation/Sensor Models/Power Meter Stub ==
  In1 : Inport
  Out1 : Outport
  wires:
  In1/1 -> Out1/1

== system MCVT_Integration/Sys3 - Power System/Power Generation Subsystem1/Nuclear Power Generation/SP-100 Power Generation/	Power Generation/Sensor Models/Thermocouple Mode Stub ==
  In1 : Inport
  In2 : Inport  Port=2
  "MAX31856 Precision Thermocouple to Digital Converter with Linearization " : Subsystem
  Omega XMO S-type Thermocouple : Subsystem
  Estimated Tf : Outport
  Cyber : Outport  Port=2
  wires:
  "MAX31856 Precision Thermocouple to Digital Converter with Linearization "/2(Cyber Output) -> Cyber/1
  In2/1 -> "MAX31856 Precision Thermocouple to Digital Converter with Linearization "/2(External Cold Junction Measurement)
  "MAX31856 Precision Thermocouple to Digital Converter with Linearization "/1(Estimated Process Temperature) -> Estimated Tf/1
  In1/1 -> Omega XMO S-type Thermocouple/1(Actual Temperature)
  Omega XMO S-type Thermocouple/1(microvolts) -> "MAX31856 Precision Thermocouple to Digital Converter with Linearization "/1(Thermocouple Measurement)

== system MCVT_Integration/Sys3 - Power System/Power Generation Subsystem1/Nuclear Power Generation/SP-100 Power Generation/	Power Generation/Sensor Models/Thermocouple Model Stub ==
  In1 : Inport
  In2 : Inport  Port=2
  "MAX31856 Precision Thermocouple to Digital Converter with Linearization " : Subsystem
  Omega XMO S-type Thermocouple : Subsystem
  Estimated Tb1 : Outport
  Cyber : Outport  Port=2
  wires:
  Omega XMO S-type Thermocouple/1(microvolts) -> "MAX31856 Precision Thermocouple to Digital Converter with Linearization "/1(Thermocouple Measurement)
  In2/1 -> "MAX31856 Precision Thermocouple to Digital Converter with Linearization "/2(External Cold Junction Measurement)
  "MAX31856 Precision Thermocouple to Digital Converter with Linearization "/2(Cyber Output) -> Cyber/1
  In1/1 -> Omega XMO S-type Thermocouple/1(Actual Temperature)
  "MAX31856 Precision Thermocouple to Digital Converter with Linearization "/1(Estimated Process Temperature) -> Estimated Tb1/1

== system MCVT_Integration/Sys3 - Power System/Power Generation Subsystem1/Nuclear Power Generation/SP-100 Power Generation/	Power Generation/Sensor Models/Thermocouple Model2 Stub ==
  In1 : Inport
  In2 : Inport  Port=2
  "MAX31856 Precision Thermocouple to Digital Converter with Linearization " : Subsystem
  Omega XMO S-type Thermocouple : Subsystem
  Estimated Tb2 : Outport
  Cyber : Outport  Port=2
  wires:
  Omega XMO S-type Thermocouple/1(microvolts) -> "MAX31856 Precision Thermocouple to Digital Converter with Linearization "/1(Thermocouple Measurement)
  In2/1 -> "MAX31856 Precision Thermocouple to Digital Converter with Linearization "/2(External Cold Junction Measurement)
  "MAX31856 Precision Thermocouple to Digital Converter with Linearization "/2(Cyber Output) -> Cyber/1
  In1/1 -> Omega XMO S-type Thermocouple/1(Actual Temperature)
  "MAX31856 Precision Thermocouple to Digital Converter with Linearization "/1(Estimated Process Temperature) -> Estimated Tb2/1

== system MCVT_Integration/Sys3 - Power System/Power Generation Subsystem1/Nuclear Power Generation/SP-100 Power Generation/	Power Generation/Sensor Models/Thermocouple Model3 Stub ==
  In1 : Inport
  In2 : Inport  Port=2
  "MAX31856 Precision Thermocouple to Digital Converter with Linearization " : Subsystem
  Omega XMO S-type Thermocouple : Subsystem
  Estimated Tc1 : Outport
  Cyber : Outport  Port=2
  wires:
  Omega XMO S-type Thermocouple/1(microvolts) -> "MAX31856 Precision Thermocouple to Digital Converter with Linearization "/1(Thermocouple Measurement)
  In2/1 -> "MAX31856 Precision Thermocouple to Digital Converter with Linearization "/2(External Cold Junction Measurement)
  "MAX31856 Precision Thermocouple to Digital Converter with Linearization "/2(Cyber Output) -> Cyber/1
  In1/1 -> Omega XMO S-type Thermocouple/1(Actual Temperature)
  "MAX31856 Precision Thermocouple to Digital Converter with Linearization "/1(Estimated Process Temperature) -> Estimated Tc1/1

== system MCVT_Integration/Sys3 - Power System/Power Generation Subsystem1/Nuclear Power Generation/SP-100 Power Generation/	Power Generation/Sensor Models/Thermocouple Model4 Stub ==
  In1 : Inport
  In2 : Inport  Port=2
  "MAX31856 Precision Thermocouple to Digital Converter with Linearization " : Subsystem
  Omega XMO S-type Thermocouple : Subsystem
  Estimated Tc2 : Outport
  Cyber : Outport  Port=2
  wires:
  Omega XMO S-type Thermocouple/1(microvolts) -> "MAX31856 Precision Thermocouple to Digital Converter with Linearization "/1(Thermocouple Measurement)
  In2/1 -> "MAX31856 Precision Thermocouple to Digital Converter with Linearization "/2(External Cold Junction Measurement)
  "MAX31856 Precision Thermocouple to Digital Converter with Linearization "/2(Cyber Output) -> Cyber/1
  In1/1 -> Omega XMO S-type Thermocouple/1(Actual Temperature)
  "MAX31856 Precision Thermocouple to Digital Converter with Linearization "/1(Estimated Process Temperature) -> Estimated Tc2/1

== system MCVT_Integration/Sys3 - Power System/Power Generation Subsystem1/Nuclear Power Generation/SP-100 Power Generation/	Power Generation/Sensor Models/block ==
  y_process_scale : Inport
  Power Out : Inport  Port=2
  Tc1_lower : Inport  Port=3
  Bus Creator : BusCreator  Inputs=6
  MATLAB Function2 : MATLAB Function  TreatAsAtomicUnit=on
  Bus : Outport
  wires:
  MATLAB Function2/3(y_Tb2) -> Bus Creator/3  "Tb2 Primary Coolant Outlet Temperature"
  Tc1_lower/1 -> Bus Creator/6  "Tc1 Secondary Coolant Inlet Temperature"
  MATLAB Function2/1(y_Tf) -> Bus Creator/1  "TF Fuel Temperature"
  Power Out/1 -> Bus Creator/5  "power_out"
  MATLAB Function2/2(y_Tb1) -> Bus Creator/2  "Tb1 Primary Coolant Inlet Temperature"
  y_process_scale/1 -> MATLAB Function2/1(y_process_scale_lower)
  MATLAB Function2/4(y_Tc2) -> Bus Creator/4  "Tc2 Secondary Coolant Outlet Temperature"
  Bus Creator/1 -> Bus/1

== system MCVT_Integration/Sys3 - Power System/Power Generation Subsystem1/Nuclear Power Generation/SP-100 Power Generation/FDD Iterative Online Residual Analysis1/ Digital Twin of SP100/Residual Generation ==
  Physical Output SP100 : Inport
  power nominal (kW) : Inport  Port=2
  Sub3_In_Intervention : Inport  Port=3
  Compare to Nominal : Subsystem
  Constant : Constant  Value=nuclear_power_toggle
  Constant1 : Constant  Value=battery_power_toggle
  FDD_toggle : MATLAB Function  TreatAsAtomicUnit=on
  Cyber Output : Outport
  wires:
  power nominal (kW)/1 -> Compare to Nominal/2(power nominal)
  Physical Output SP100/1 -> Compare to Nominal/1(Physical Output SP100)
  Sub3_In_Intervention/1 -> Compare to Nominal/3(Sub3_In_Intervention)
  Compare to Nominal/1(FDD) -> FDD_toggle/1(FDD_signal)  "nuc_power_FDD"
  FDD_toggle/1(FDD_signal_sent) -> Cyber Output/1  "nuc_power_FDD_post"
  [nuc_toggle] -> FDD_toggle/2(nuclear_power_toggle)
  [batt_toggle] -> FDD_toggle/3(battery_power_toggle)
  Constant/1 -> [nuc_toggle]
  Constant1/1 -> [batt_toggle]

== system MCVT_Integration/Sys2 - Structural System/Sys2_Structural_System/Fault Detection and Diagnosis (FDD) with Binary Classifer/Subsystem/Enabled Subsystem3/Pattern Recognition Neural Network1/Layer 1/Delays 1 ==
  "p{1}" : Inport  PortDimensions=45  SampleTime=0.001
      mask Display: e = 0.06;c1 = [120 230 180]/255;c2 = ([120 230 180]+0)/(256+128);c3 = ([120 230 180]+128)/(256+128);patch([0 1 1 0],[0 0 1 1],c1);patch([0 1 1 1-e 1-e 0+e],[0 0 1 1-e 0+e 0+e],c2);patch([0 0 1 1-e 0+e 0+e],[0 1 1 1-e 1-e 0+e],c3);plot([0 1 1 0 0],[0 0 1 1 0]);
  mux : Mux  Inputs=1
      mask Display: e = 0.06;c1 = [120 150 230]/255;c2 = ([120 150 230]+0)/383;c3 = ([120 150 230]+128)/383;patch([0 1 1 0],[0 0 1 1],c1);patch([0 1 1 1-e 1-e 0+e],[0 0 1 1-e 0+e 0+e],c2);patch([0 0 1 1-e 0+e 0+e],[0 1 1 1-e 1-e 0+e],c3);plot([0 1 1 0 0],[0 0 1 1 0]);disp('Mux')
  "pd{1,1}" : Outport
      mask Display: e = 0.06;c1 = [120 230 180]/255;c2 = ([120 230 180]+0)/(256+128);c3 = ([120 230 180]+128)/(256+128);patch([0 1 1 0],[0 0 1 1],c1);patch([0 1 1 1-e 1-e 0+e],[0 0 1 1-e 0+e 0+e],c2);patch([0 0 1 1-e 0+e 0+e],[0 1 1 1-e 1-e 0+e],c3);plot([0 1 1 0 0],[0 0 1 1 0]);
  wires:
  "p{1}"/1 -> mux/1
  mux/1 -> "pd{1,1}"/1
